simulink model "AuxClimaCtlMgr"  (R2022b) (continued)
== system AuxClimaCtlMgr == (continued)
  VFCPrkDrvgClimabyFragAct_VFCPrkDrvgClimabyFragAct : Outport  Port=66  OutDataTypeStr=Boolean  PortDimensions=1
  wires:
  AirClngSts_AirClngSts/1 -> AuxClimaCtlMgr_Runnable_sys/1(AirClngSts_AirClngSts_read)
  AirFragCh1AvlTi_AirFragCh1AvlTi/1 -> AuxClimaCtlMgr_Runnable_sys/2(AirFragCh1AvlTi_AirFragCh1AvlTi_read)
  AirFragCh1RunngSts_AirFragCh1RunngSts/1 -> AuxClimaCtlMgr_Runnable_sys/3(AirFragCh1RunngSts_AirFragCh1RunngSts_read)
  AirFragCh1Type_AirFragCh1Type/1 -> AuxClimaCtlMgr_Runnable_sys/4(AirFragCh1Type_AirFragCh1Type_read)
  AirFragCh2AvlTi_AirFragCh2AvlTi/1 -> AuxClimaCtlMgr_Runnable_sys/5(AirFragCh2AvlTi_AirFragCh2AvlTi_read)
  AirFragCh2RunngSts_AirFragCh2RunngSts/1 -> AuxClimaCtlMgr_Runnable_sys/6(AirFragCh2RunngSts_AirFragCh2RunngSts_read)
  AirFragCh2Type_AirFragCh2Type/1 -> AuxClimaCtlMgr_Runnable_sys/7(AirFragCh2Type_AirFragCh2Type_read)
  AirFragCh3AvlTi_AirFragCh3AvlTi/1 -> AuxClimaCtlMgr_Runnable_sys/8(AirFragCh3AvlTi_AirFragCh3AvlTi_read)
  AirFragCh3RunngSts_AirFragCh3RunngSts/1 -> AuxClimaCtlMgr_Runnable_sys/9(AirFragCh3RunngSts_AirFragCh3RunngSts_read)
  AirFragCh3Type_AirFragCh3Type/1 -> AuxClimaCtlMgr_Runnable_sys/10(AirFragCh3Type_AirFragCh3Type_read)
  AirFragCh4AvlTi_AirFragCh4AvlTi/1 -> AuxClimaCtlMgr_Runnable_sys/11(AirFragCh4AvlTi_AirFragCh4AvlTi_read)
  AirFragCh4RunngSts_AirFragCh4RunngSts/1 -> AuxClimaCtlMgr_Runnable_sys/12(AirFragCh4RunngSts_AirFragCh4RunngSts_read)
  AirFragCh4Type_AirFragCh4Type/1 -> AuxClimaCtlMgr_Runnable_sys/13(AirFragCh4Type_AirFragCh4Type_read)
  AirFragCh5AvlTi_AirFragCh5AvlTi/1 -> AuxClimaCtlMgr_Runnable_sys/14(AirFragCh5AvlTi_AirFragCh5AvlTi_read)
  AirFragCh5RunngSts_AirFragCh5RunngSts/1 -> AuxClimaCtlMgr_Runnable_sys/15(AirFragCh5RunngSts_AirFragCh5RunngSts_read)
  AirFragCh5Type_AirFragCh5Type/1 -> AuxClimaCtlMgr_Runnable_sys/16(AirFragCh5Type_AirFragCh5Type_read)
  AirFragChgSts_AirFragChgSts/1 -> AuxClimaCtlMgr_Runnable_sys/17(AirFragChgSts_AirFragChgSts_read)
  AirFragErrSts_AirFragErrSts/1 -> AuxClimaCtlMgr_Runnable_sys/18(AirFragErrSts_AirFragErrSts_read)
  AirFragInitSts_AirFragInitSts/1 -> AuxClimaCtlMgr_Runnable_sys/19(AirFragInitSts_AirFragInitSts_read)
  AirFragLvlRspn_AirFragLvlRspn/1 -> AuxClimaCtlMgr_Runnable_sys/20(AirFragLvlRspn_AirFragLvlRspn_read)
  AmbTEstimd_Ngcc_AmbTEstimd/1 -> AuxClimaCtlMgr_Runnable_sys/21(AmbTEstimd_Ngcc_AmbTEstimd_read)
  ClimaCfgForAirClng1_ClimaCfgForAirClng1/1 -> AuxClimaCtlMgr_Runnable_sys/22(ClimaCfgForAirClng1_ClimaCfgForAirClng1_read)
  ClimaCfgForAirFrag_ClimaCfgForAirFrag/1 -> AuxClimaCtlMgr_Runnable_sys/23(ClimaCfgForAirFrag_ClimaCfgForAirFrag_read)
  ClimaCfgForCds1_ClimaCfgForCds1/1 -> AuxClimaCtlMgr_Runnable_sys/24(ClimaCfgForCds1_ClimaCfgForCds1_read)
  ClimaCfgForVehElec1_ClimaCfgForVehElec1/1 -> AuxClimaCtlMgr_Runnable_sys/25(ClimaCfgForVehElec1_ClimaCfgForVehElec1_read)
  DrvrPfmncAlrmReq_DrvrPfmncAlrmReq/1 -> AuxClimaCtlMgr_Runnable_sys/26(DrvrPfmncAlrmReq_DrvrPfmncAlrmReq_read)
  DrvrPfmncAlrmReqFromDHU_DrvrPfmncAlrmReqFromDHU/1 -> AuxClimaCtlMgr_Runnable_sys/27(DrvrPfmncAlrmReqFromDHU_DrvrPfmncAlrmReqFromDHU_read)
  FragRefreshPopUpSucs_FragRefreshPopUpSucs/1 -> AuxClimaCtlMgr_Runnable_sys/28(FragRefreshPopUpSucs_FragRefreshPopUpSucs_read)
  FragRefrshAutSetg_FragRefrshAutSetg/1 -> AuxClimaCtlMgr_Runnable_sys/29(FragRefrshAutSetg_FragRefrshAutSetg_read)
  FrntHvacBlowerSts_FrntHvacBlowerSts/1 -> AuxClimaCtlMgr_Runnable_sys/30(FrntHvacBlowerSts_FrntHvacBlowerSts_read)
  HmiFragraModReq_HmiFragraModReq/1 -> AuxClimaCtlMgr_Runnable_sys/31(HmiFragraModReq_HmiFragraModReq_read)
  HmiPopUpResp_HmiPopUpResp/1 -> AuxClimaCtlMgr_Runnable_sys/32(HmiPopUpResp_HmiPopUpResp_read)
  InCarCo2HighWarnSucs_InCarCo2HighWarnSucs/1 -> AuxClimaCtlMgr_Runnable_sys/33(InCarCo2HighWarnSucs_InCarCo2HighWarnSucs_read)
  IntCo2CircElecErr_IntCo2CircElecErr/1 -> AuxClimaCtlMgr_Runnable_sys/34(IntCo2CircElecErr_IntCo2CircElecErr_read)
  IntCo2Conc_IntCo2Conc/1 -> AuxClimaCtlMgr_Runnable_sys/35(IntCo2Conc_IntCo2Conc_read)
  IntCo2fromExsrc_IntCo2fromExsrc/1 -> AuxClimaCtlMgr_Runnable_sys/36(IntCo2fromExsrc_IntCo2fromExsrc_read)
  IntCo2HvErr_IntCo2HvErr/1 -> AuxClimaCtlMgr_Runnable_sys/37(IntCo2HvErr_IntCo2HvErr_read)
  IntCo2LvErr_IntCo2LvErr/1 -> AuxClimaCtlMgr_Runnable_sys/38(IntCo2LvErr_IntCo2LvErr_read)
  IntCo2Lvl_IntCo2Lvl/1 -> AuxClimaCtlMgr_Runnable_sys/39(IntCo2Lvl_IntCo2Lvl_read)
  IntCo2RunMod_IntCo2RunMod/1 -> AuxClimaCtlMgr_Runnable_sys/40(IntCo2RunMod_IntCo2RunMod_read)
  IntCo2RunSts_IntCo2RunSts/1 -> AuxClimaCtlMgr_Runnable_sys/41(IntCo2RunSts_IntCo2RunSts_read)
  PwrAllwdForAirFrag_PwrAllwdForAirFrag/1 -> AuxClimaCtlMgr_Runnable_sys/42(PwrAllwdForAirFrag_PwrAllwdForAirFrag_read)
  VehModMngtGlbSafe1_Ngcc_VehModMngtGlbSafe1/1 -> AuxClimaCtlMgr_Runnable_sys/43(VehModMngtGlbSafe1_Ngcc_VehModMngtGlbSafe1_read)
  VehSunLoadTot_VehSunLoadTot/1 -> AuxClimaCtlMgr_Runnable_sys/44(VehSunLoadTot_VehSunLoadTot_read)
  CnctSrvFragChRatReq_CnctSrvFragChRatReq/1 -> AuxClimaCtlMgr_Runnable_sys/45(CnctSrvFragChRatReq_CnctSrvFragChRatReq_read)
  CnctSrvFragChRatReq_CnctSrvFragChRatReq_IsUpdated/1 -> AuxClimaCtlMgr_Runnable_sys/46(CnctSrvFragChRatReq_CnctSrvFragChRatReq_IsUpdated_read)
  CnctSrvFragLvlReq_CnctSrvFragLvlReq/1 -> AuxClimaCtlMgr_Runnable_sys/47(CnctSrvFragLvlReq_CnctSrvFragLvlReq_read)
  CnctSrvFragLvlReq_CnctSrvFragLvlReq_IsUpdated/1 -> AuxClimaCtlMgr_Runnable_sys/48(CnctSrvFragLvlReq_CnctSrvFragLvlReq_IsUpdated_read)
  HmiFragraChRatReq_HmiFragraChRatReq/1 -> AuxClimaCtlMgr_Runnable_sys/49(HmiFragraChRatReq_HmiFragraChRatReq_read)
  HmiFragraChRatReq_HmiFragraChRatReq_IsUpdated/1 -> AuxClimaCtlMgr_Runnable_sys/50(HmiFragraChRatReq_HmiFragraChRatReq_IsUpdated_read)
  HmiFragraLvlReq_HmiFragraLvlReq/1 -> AuxClimaCtlMgr_Runnable_sys/51(HmiFragraLvlReq_HmiFragraLvlReq_read)
  HmiFragraLvlReq_HmiFragraLvlReq_IsUpdated/1 -> AuxClimaCtlMgr_Runnable_sys/52(HmiFragraLvlReq_HmiFragraLvlReq_IsUpdated_read)
  ReqFragLvlTelm_ReqFragLvlTelm/1 -> AuxClimaCtlMgr_Runnable_sys/53(ReqFragLvlTelm_ReqFragLvlTelm_read)
  ReqFragLvlTelm_ReqFragLvlTelm_IsUpdated/1 -> AuxClimaCtlMgr_Runnable_sys/54(ReqFragLvlTelm_ReqFragLvlTelm_IsUpdated_read)
  TelmAirFragTasteReq_TelmAirFragTasteReq/1 -> AuxClimaCtlMgr_Runnable_sys/55(TelmAirFragTasteReq_TelmAirFragTasteReq_read)
  TelmAirFragTasteReq_TelmAirFragTasteReq_IsUpdated/1 -> AuxClimaCtlMgr_Runnable_sys/56(TelmAirFragTasteReq_TelmAirFragTasteReq_IsUpdated_read)
  AACAirClngStsNgcc_AACAirClngStsNgcc/1 -> AuxClimaCtlMgr_Runnable_sys/57(AACAirClngStsNgcc_AACAirClngStsNgcc_read)
  AFUFragraCh1AvlTiPrecNgcc_AFUFragraCh1AvlTiPrecNgcc/1 -> AuxClimaCtlMgr_Runnable_sys/58(AFUFragraCh1AvlTiPrecNgcc_AFUFragraCh1AvlTiPrecNgcc_read)
  AFUFragraCh1AvlTiPrecNgcc_AFUFragraCh1AvlTiPrecNgcc_IsUpdated/1 -> AuxClimaCtlMgr_Runnable_sys/59(AFUFragraCh1AvlTiPrecNgcc_AFUFragraCh1AvlTiPrecNgcc_IsUpdated_read)
  AFUFragraCh2AvlTiPrecNgcc_AFUFragraCh2AvlTiPrecNgcc/1 -> AuxClimaCtlMgr_Runnable_sys/60(AFUFragraCh2AvlTiPrecNgcc_AFUFragraCh2AvlTiPrecNgcc_read)
  AFUFragraCh2AvlTiPrecNgcc_AFUFragraCh2AvlTiPrecNgcc_IsUpdated/1 -> AuxClimaCtlMgr_Runnable_sys/61(AFUFragraCh2AvlTiPrecNgcc_AFUFragraCh2AvlTiPrecNgcc_IsUpdated_read)
  AFUFragraCh3AvlTiPrecNgcc_AFUFragraCh3AvlTiPrecNgcc/1 -> AuxClimaCtlMgr_Runnable_sys/62(AFUFragraCh3AvlTiPrecNgcc_AFUFragraCh3AvlTiPrecNgcc_read)
  AFUFragraCh3AvlTiPrecNgcc_AFUFragraCh3AvlTiPrecNgcc_IsUpdated/1 -> AuxClimaCtlMgr_Runnable_sys/63(AFUFragraCh3AvlTiPrecNgcc_AFUFragraCh3AvlTiPrecNgcc_IsUpdated_read)
  AFUFragraCh4AvlTiPrecNgcc_AFUFragraCh4AvlTiPrecNgcc/1 -> AuxClimaCtlMgr_Runnable_sys/64(AFUFragraCh4AvlTiPrecNgcc_AFUFragraCh4AvlTiPrecNgcc_read)
  AFUFragraCh4AvlTiPrecNgcc_AFUFragraCh4AvlTiPrecNgcc_IsUpdated/1 -> AuxClimaCtlMgr_Runnable_sys/65(AFUFragraCh4AvlTiPrecNgcc_AFUFragraCh4AvlTiPrecNgcc_IsUpdated_read)
  AFUFragraCh5AvlTiPrecNgcc_AFUFragraCh5AvlTiPrecNgcc/1 -> AuxClimaCtlMgr_Runnable_sys/66(AFUFragraCh5AvlTiPrecNgcc_AFUFragraCh5AvlTiPrecNgcc_read)
  AFUFragraCh5AvlTiPrecNgcc_AFUFragraCh5AvlTiPrecNgcc_IsUpdated/1 -> AuxClimaCtlMgr_Runnable_sys/67(AFUFragraCh5AvlTiPrecNgcc_AFUFragraCh5AvlTiPrecNgcc_IsUpdated_read)
  AirClngReq_AirClngReq/1 -> AuxClimaCtlMgr_Runnable_sys/68(AirClngReq_AirClngReq_read)
  AirClngReq_AirClngReq_IsUpdated/1 -> AuxClimaCtlMgr_Runnable_sys/69(AirClngReq_AirClngReq_IsUpdated_read)
  AirFragCh1OvrdDyNgcc_AirFragCh1OvrdDyNgcc/1 -> AuxClimaCtlMgr_Runnable_sys/70(AirFragCh1OvrdDyNgcc_AirFragCh1OvrdDyNgcc_read)
  AirFragCh2OvrdDyNgcc_AirFragCh2OvrdDyNgcc/1 -> AuxClimaCtlMgr_Runnable_sys/71(AirFragCh2OvrdDyNgcc_AirFragCh2OvrdDyNgcc_read)
  AirFragCh3OvrdDyNgcc_AirFragCh3OvrdDyNgcc/1 -> AuxClimaCtlMgr_Runnable_sys/72(AirFragCh3OvrdDyNgcc_AirFragCh3OvrdDyNgcc_read)
  AirFragCh4OvrdDyNgcc_AirFragCh4OvrdDyNgcc/1 -> AuxClimaCtlMgr_Runnable_sys/73(AirFragCh4OvrdDyNgcc_AirFragCh4OvrdDyNgcc_read)
  AirFragCh5OvrdDyNgcc_AirFragCh5OvrdDyNgcc/1 -> AuxClimaCtlMgr_Runnable_sys/74(AirFragCh5OvrdDyNgcc_AirFragCh5OvrdDyNgcc_read)
  AirSrcStsFbNgcc_AirSrcStsFbNgcc/1 -> AuxClimaCtlMgr_Runnable_sys/75(AirSrcStsFbNgcc_AirSrcStsFbNgcc_read)
  AltitudeInfromNgcc_AltitudeInfromNgcc/1 -> AuxClimaCtlMgr_Runnable_sys/76(AltitudeInfromNgcc_AltitudeInfromNgcc_read)
  ClimaCfgForAAC_ClimaCfgForAAC/1 -> AuxClimaCtlMgr_Runnable_sys/77(ClimaCfgForAAC_ClimaCfgForAAC_read)
  ClimaCfgForAirClnrAdv1_ClimaCfgForAirClnrAdv1/1 -> AuxClimaCtlMgr_Runnable_sys/78(ClimaCfgForAirClnrAdv1_ClimaCfgForAirClnrAdv1_read)
  ClimaCfgForCabinOxgenSupply_ClimaCfgForCabinOxgenSupply/1 -> AuxClimaCtlMgr_Runnable_sys/79(ClimaCfgForCabinOxgenSupply_ClimaCfgForCabinOxgenSupply_read)
  ClimaCfgForPrpsnTyp_ClimaCfgForPrpsnTyp/1 -> AuxClimaCtlMgr_Runnable_sys/80(ClimaCfgForPrpsnTyp_ClimaCfgForPrpsnTyp_read)
  ClimaCfgForRoofHatch_ClimaCfgForRoofHatch/1 -> AuxClimaCtlMgr_Runnable_sys/81(ClimaCfgForRoofHatch_ClimaCfgForRoofHatch_read)
  CmptmtOxygenConcQFNgcc_CmptmtOxygenConcQFNgcc/1 -> AuxClimaCtlMgr_Runnable_sys/82(CmptmtOxygenConcQFNgcc_CmptmtOxygenConcQFNgcc_read)
  CmptmtOxygenConcVluNgcc_CmptmtOxygenConcVluNgcc/1 -> AuxClimaCtlMgr_Runnable_sys/83(CmptmtOxygenConcVluNgcc_CmptmtOxygenConcVluNgcc_read)
  DispHvBattLvlOfChrgNgcc_DispHvBattLvlOfChrgNgcc/1 -> AuxClimaCtlMgr_Runnable_sys/84(DispHvBattLvlOfChrgNgcc_DispHvBattLvlOfChrgNgcc_read)
  DoorDrvrReSts_DoorDrvrReSts/1 -> AuxClimaCtlMgr_Runnable_sys/85(DoorDrvrReSts_DoorDrvrReSts_read)
  DoorDrvrSts_Ngcc_DoorDrvrSts/1 -> AuxClimaCtlMgr_Runnable_sys/86(DoorDrvrSts_Ngcc_DoorDrvrSts_read)
  DoorPassReSts_DoorPassReSts/1 -> AuxClimaCtlMgr_Runnable_sys/87(DoorPassReSts_DoorPassReSts_read)
  DoorPassSts_Ngcc_DoorPassSts/1 -> AuxClimaCtlMgr_Runnable_sys/88(DoorPassSts_Ngcc_DoorPassSts_read)
  DrvrVentLeClsdFlag_DrvrVentLeClsdFlag/1 -> AuxClimaCtlMgr_Runnable_sys/89(DrvrVentLeClsdFlag_DrvrVentLeClsdFlag_read)
  DrvrVentRiClsdFlag_DrvrVentRiClsdFlag/1 -> AuxClimaCtlMgr_Runnable_sys/90(DrvrVentRiClsdFlag_DrvrVentRiClsdFlag_read)
  HmiAutOxySetNgcc_HmiAutOxySetNgcc/1 -> AuxClimaCtlMgr_Runnable_sys/91(HmiAutOxySetNgcc_HmiAutOxySetNgcc_read)
  HmiOxyinCarSwtNgcc_HmiOxyinCarSwtNgcc/1 -> AuxClimaCtlMgr_Runnable_sys/92(HmiOxyinCarSwtNgcc_HmiOxyinCarSwtNgcc_read)
  HvacRecircAct_Ngcc_HvacRecircAct/1 -> AuxClimaCtlMgr_Runnable_sys/93(HvacRecircAct_Ngcc_HvacRecircAct_read)
  HvSysRlySts_HvSysRlySts/1 -> AuxClimaCtlMgr_Runnable_sys/94(HvSysRlySts_HvSysRlySts_read)
  InflateAirSrcSwtNgcc_InflateAirSrcSwtNgcc/1 -> AuxClimaCtlMgr_Runnable_sys/95(InflateAirSrcSwtNgcc_InflateAirSrcSwtNgcc_read)
  OGMAirSrcReq_OGMAirSrcReq/1 -> AuxClimaCtlMgr_Runnable_sys/96(OGMAirSrcReq_OGMAirSrcReq_read)
  OGMAvlSts_OGMAvlSts/1 -> AuxClimaCtlMgr_Runnable_sys/97(OGMAvlSts_OGMAvlSts_read)
  OGMIntErrNgcc_OGMIntErrNgcc/1 -> AuxClimaCtlMgr_Runnable_sys/98(OGMIntErrNgcc_OGMIntErrNgcc_read)
  OGMOvrVoltRangErrNgcc_OGMOvrVoltRangErrNgcc/1 -> AuxClimaCtlMgr_Runnable_sys/99(OGMOvrVoltRangErrNgcc_OGMOvrVoltRangErrNgcc_read)
  OGMReq_OGMReq/1 -> AuxClimaCtlMgr_Runnable_sys/100(OGMReq_OGMReq_read)
  OGMTempErrNgcc_OGMTempErrNgcc/1 -> AuxClimaCtlMgr_Runnable_sys/101(OGMTempErrNgcc_OGMTempErrNgcc_read)
  OGMUndVoltRangErrNgcc_OGMUndVoltRangErrNgcc/1 -> AuxClimaCtlMgr_Runnable_sys/102(OGMUndVoltRangErrNgcc_OGMUndVoltRangErrNgcc_read)
  OxygenConcSnsrElecErrNgcc_OxygenConcSnsrElecErrNgcc/1 -> AuxClimaCtlMgr_Runnable_sys/103(OxygenConcSnsrElecErrNgcc_OxygenConcSnsrElecErrNgcc_read)
  OxygenConcSnsrIntErrNgcc_OxygenConcSnsrIntErrNgcc/1 -> AuxClimaCtlMgr_Runnable_sys/104(OxygenConcSnsrIntErrNgcc_OxygenConcSnsrIntErrNgcc_read)
  OxygenConcSnsrOvrVoltRangErrNgcc_OxygenConcSnsrOvrVoltRangErrNgcc/1 -> AuxClimaCtlMgr_Runnable_sys/105(OxygenConcSnsrOvrVoltRangErrNgcc_OxygenConcSnsrOvrVoltRangErrNgcc_read)
  OxygenConcSnsrStsNgcc_OxygenConcSnsrStsNgcc/1 -> AuxClimaCtlMgr_Runnable_sys/106(OxygenConcSnsrStsNgcc_OxygenConcSnsrStsNgcc_read)
  OxygenConcSnsrTempErrNgcc_OxygenConcSnsrTempErrNgcc/1 -> AuxClimaCtlMgr_Runnable_sys/107(OxygenConcSnsrTempErrNgcc_OxygenConcSnsrTempErrNgcc_read)
  OxygenConcSnsrUndVoltRangErrNgcc_OxygenConcSnsrUndVoltRangErrNgcc/1 -> AuxClimaCtlMgr_Runnable_sys/108(OxygenConcSnsrUndVoltRangErrNgcc_OxygenConcSnsrUndVoltRangErrNgcc_read)
  OxygenGenrErrInfoNgcc_OxygenGenrErrInfoNgcc/1 -> AuxClimaCtlMgr_Runnable_sys/109(OxygenGenrErrInfoNgcc_OxygenGenrErrInfoNgcc_read)
  OxygenSplyAvlStsNgcc_OxygenSplyAvlStsNgcc/1 -> AuxClimaCtlMgr_Runnable_sys/110(OxygenSplyAvlStsNgcc_OxygenSplyAvlStsNgcc_read)
  OxygenSplyErrNgcc_OxygenSplyErrNgcc/1 -> AuxClimaCtlMgr_Runnable_sys/111(OxygenSplyErrNgcc_OxygenSplyErrNgcc_read)
  OxygenSplyPwrAllwdNgcc_OxygenSplyPwrAllwdNgcc/1 -> AuxClimaCtlMgr_Runnable_sys/112(OxygenSplyPwrAllwdNgcc_OxygenSplyPwrAllwdNgcc_read)
  PassVentLeClsdFlag_PassVentLeClsdFlag/1 -> AuxClimaCtlMgr_Runnable_sys/113(PassVentLeClsdFlag_PassVentLeClsdFlag_read)
  PassVentRiClsdFlag_PassVentRiClsdFlag/1 -> AuxClimaCtlMgr_Runnable_sys/114(PassVentRiClsdFlag_PassVentRiClsdFlag_read)
  RemOxygenSplyActv_RemOxygenSplyActv/1 -> AuxClimaCtlMgr_Runnable_sys/115(RemOxygenSplyActv_RemOxygenSplyActv_read)
  SunRoofPosnSts_Ngcc_SunRoofPosnSts/1 -> AuxClimaCtlMgr_Runnable_sys/116(SunRoofPosnSts_Ngcc_SunRoofPosnSts_read)
  TelmOxygenValReqNgcc_TelmOxygenValReqNgcc/1 -> AuxClimaCtlMgr_Runnable_sys/117(TelmOxygenValReqNgcc_TelmOxygenValReqNgcc_read)
  TiAndDateIndcn_TiAndDateIndcn/1 -> AuxClimaCtlMgr_Runnable_sys/118(TiAndDateIndcn_TiAndDateIndcn_read)
  TrSts_Ngcc_TrSts/1 -> AuxClimaCtlMgr_Runnable_sys/119(TrSts_Ngcc_TrSts_read)
  TrSts_Ngcc_TrSts_ErrorStatus/1 -> AuxClimaCtlMgr_Runnable_sys/120(TrSts_Ngcc_TrSts_ErrorStatus_read)
  WinPosnStsAtDrvrReNgcc_WinPosnStsAtDrvrReNgcc/1 -> AuxClimaCtlMgr_Runnable_sys/121(WinPosnStsAtDrvrReNgcc_WinPosnStsAtDrvrReNgcc_read)
  WinPosnStsAtPassReNgcc_WinPosnStsAtPassRe/1 -> AuxClimaCtlMgr_Runnable_sys/122(WinPosnStsAtPassReNgcc_WinPosnStsAtPassRe_read)
  WinPosnStsDrv_WinPosnStsAtDrv/1 -> AuxClimaCtlMgr_Runnable_sys/123(WinPosnStsDrv_WinPosnStsAtDrv_read)
  WinPosnStsPass_WinPosnStsAtPass/1 -> AuxClimaCtlMgr_Runnable_sys/124(WinPosnStsPass_WinPosnStsAtPass_read)
  AuxClimaCtlMgr_Runnable_sys/1(AACAirClngActnNgcc_AACAirClngActnNgcc_write) -> AACAirClngActnNgcc_AACAirClngActnNgcc/1
  AuxClimaCtlMgr_Runnable_sys/2(AirSrcReqNgcc_AirSrcReqNgcc_write) -> AirSrcReqNgcc_AirSrcReqNgcc/1
  AuxClimaCtlMgr_Runnable_sys/3(AutOxygenSplyStsFrmClimaNgcc_AutOxygenSplyStsFrmClimaNgcc_write) -> AutOxygenSplyStsFrmClimaNgcc_AutOxygenSplyStsFrmClimaNgcc/1
  AuxClimaCtlMgr_Runnable_sys/4(CcmAACAirClngStsNgcc_CcmAACAirClngStsNgcc_write) -> CcmAACAirClngStsNgcc_CcmAACAirClngStsNgcc/1
  AuxClimaCtlMgr_Runnable_sys/5(CCMFragCh1OvrdDyNgcc_CCMFragCh1OvrdDyNgcc_write) -> CCMFragCh1OvrdDyNgcc_CCMFragCh1OvrdDyNgcc/1
  AuxClimaCtlMgr_Runnable_sys/6(CCMFragCh2OvrdDyNgcc_CCMFragCh2OvrdDyNgcc_write) -> CCMFragCh2OvrdDyNgcc_CCMFragCh2OvrdDyNgcc/1
  AuxClimaCtlMgr_Runnable_sys/7(CCMFragCh3OvrdDyNgcc_CCMFragCh3OvrdDyNgcc_write) -> CCMFragCh3OvrdDyNgcc_CCMFragCh3OvrdDyNgcc/1
  AuxClimaCtlMgr_Runnable_sys/8(CCMFragCh4OvrdDyNgcc_CCMFragCh4OvrdDyNgcc_write) -> CCMFragCh4OvrdDyNgcc_CCMFragCh4OvrdDyNgcc/1
  AuxClimaCtlMgr_Runnable_sys/9(CCMFragCh5OvrdDyNgcc_CCMFragCh5OvrdDyNgcc_write) -> CCMFragCh5OvrdDyNgcc_CCMFragCh5OvrdDyNgcc/1
  AuxClimaCtlMgr_Runnable_sys/10(CCMFragraCh1AvlTiPrecNgcc_CCMFragraCh1AvlTiPrecNgcc_write) -> CCMFragraCh1AvlTiPrecNgcc_CCMFragraCh1AvlTiPrecNgcc/1
  AuxClimaCtlMgr_Runnable_sys/11(CCMFragraCh2AvlTiPrecNgcc_CCMFragraCh2AvlTiPrecNgcc_write) -> CCMFragraCh2AvlTiPrecNgcc_CCMFragraCh2AvlTiPrecNgcc/1
  AuxClimaCtlMgr_Runnable_sys/12(CCMFragraCh3AvlTiPrecNgcc_CCMFragraCh3AvlTiPrecNgcc_write) -> CCMFragraCh3AvlTiPrecNgcc_CCMFragraCh3AvlTiPrecNgcc/1
  AuxClimaCtlMgr_Runnable_sys/13(CCMFragraCh4AvlTiPrecNgcc_CCMFragraCh4AvlTiPrecNgcc_write) -> CCMFragraCh4AvlTiPrecNgcc_CCMFragraCh4AvlTiPrecNgcc/1
  AuxClimaCtlMgr_Runnable_sys/14(CCMFragraCh5AvlTiPrecNgcc_CCMFragraCh5AvlTiPrecNgcc_write) -> CCMFragraCh5AvlTiPrecNgcc_CCMFragraCh5AvlTiPrecNgcc/1
  AuxClimaCtlMgr_Runnable_sys/15(CmptmtOxygenConcLvlFrmClimaNgcc_CmptmtOxygenConcLvlFrmClimaNgcc_write) -> CmptmtOxygenConcLvlFrmClimaNgcc_CmptmtOxygenConcLvlFrmClimaNgcc/1
  AuxClimaCtlMgr_Runnable_sys/16(CmptmtOxygenConcVluFrmClimaNgcc_CmptmtOxygenConcVluFrmClimaNgcc_write) -> CmptmtOxygenConcVluFrmClimaNgcc_CmptmtOxygenConcVluFrmClimaNgcc/1
  AuxClimaCtlMgr_Runnable_sys/17(OSMRecircRatReq_OSMRecircRatReq_write) -> OSMRecircRatReq_OSMRecircRatReq/1
  AuxClimaCtlMgr_Runnable_sys/18(OxygenConcSnsrActnReqNgcc_OxygenConcSnsrActnReqNgcc_write) -> OxygenConcSnsrActnReqNgcc_OxygenConcSnsrActnReqNgcc/1
  AuxClimaCtlMgr_Runnable_sys/19(OxygenConcSnsrStsFrmClimaNgcc_OxygenConcSnsrStsFrmClimaNgcc_write) -> OxygenConcSnsrStsFrmClimaNgcc_OxygenConcSnsrStsFrmClimaNgcc/1
  AuxClimaCtlMgr_Runnable_sys/20(OxygenSplyActPwrCns_OxygenSplyActPwrCns_write) -> OxygenSplyActPwrCns_OxygenSplyActPwrCns/1
  AuxClimaCtlMgr_Runnable_sys/21(OxygenSplyAvlStsFrmClimaNgcc_OxygenSplyAvlStsFrmClimaNgcc_write) -> OxygenSplyAvlStsFrmClimaNgcc_OxygenSplyAvlStsFrmClimaNgcc/1
  AuxClimaCtlMgr_Runnable_sys/22(OxygenSplyDesPwrCns_OxygenSplyDesPwrCns_write) -> OxygenSplyDesPwrCns_OxygenSplyDesPwrCns/1
  AuxClimaCtlMgr_Runnable_sys/23(OxygenSplyErrFrmClimaNgcc_OxygenSplyErrFrmClimaNgcc_write) -> OxygenSplyErrFrmClimaNgcc_OxygenSplyErrFrmClimaNgcc/1
  AuxClimaCtlMgr_Runnable_sys/24(OxygenSplyReqFaildNgcc_OxygenSplyReqFaildNgcc_write) -> OxygenSplyReqFaildNgcc_OxygenSplyReqFaildNgcc/1
  AuxClimaCtlMgr_Runnable_sys/25(OxygenSplyReqNgcc_OxygenSplyReqNgcc_write) -> OxygenSplyReqNgcc_OxygenSplyReqNgcc/1
  AuxClimaCtlMgr_Runnable_sys/26(OxygenSplyVoiceFbNgcc_OxygenSplyVoiceFbNgcc_write) -> OxygenSplyVoiceFbNgcc_OxygenSplyVoiceFbNgcc/1
  AuxClimaCtlMgr_Runnable_sys/27(PopUpReqFromOxygenSply_PopUpReqFromOxygenSply_write) -> PopUpReqFromOxygenSply_PopUpReqFromOxygenSply/1
  AuxClimaCtlMgr_Runnable_sys/28(VFCPrkDrvgClimabyOxygenSplyCtrl_VFCPrkDrvgClimabyOxygenSplyCtrl_write) -> VFCPrkDrvgClimabyOxygenSplyCtrl_VFCPrkDrvgClimabyOxygenSplyCtrl/1
  AuxClimaCtlMgr_Runnable_sys/29(AirClngActn_AirClngActn_write) -> AirClngActn_AirClngActn/1
  AuxClimaCtlMgr_Runnable_sys/30(AirFragActEnad_AirFragActEnad_write) -> AirFragActEnad_AirFragActEnad/1
  AuxClimaCtlMgr_Runnable_sys/31(AirFragCh1_AirFragCh1_write) -> AirFragCh1_AirFragCh1/1
  AuxClimaCtlMgr_Runnable_sys/32(AirFragCh2_AirFragCh2_write) -> AirFragCh2_AirFragCh2/1
  AuxClimaCtlMgr_Runnable_sys/33(AirFragCh3_AirFragCh3_write) -> AirFragCh3_AirFragCh3/1
  AuxClimaCtlMgr_Runnable_sys/34(AirFragCh4_AirFragCh4_write) -> AirFragCh4_AirFragCh4/1
  AuxClimaCtlMgr_Runnable_sys/35(AirFragCh5_AirFragCh5_write) -> AirFragCh5_AirFragCh5/1
  AuxClimaCtlMgr_Runnable_sys/36(AirFragConcReq_AirFragConcReq_write) -> AirFragConcReq_AirFragConcReq/1
  AuxClimaCtlMgr_Runnable_sys/37(AirFragPwrCnsAct_AirFragPwrCnsAct_write) -> AirFragPwrCnsAct_AirFragPwrCnsAct/1
  AuxClimaCtlMgr_Runnable_sys/38(AirFragPwrCnsDes_AirFragPwrCnsDes_write) -> AirFragPwrCnsDes_AirFragPwrCnsDes/1
  AuxClimaCtlMgr_Runnable_sys/39(CcmAirClngSts_CcmAirClngSts_write) -> CcmAirClngSts_CcmAirClngSts/1
  AuxClimaCtlMgr_Runnable_sys/40(CcmIntCo2Conc_CcmIntCo2Conc_write) -> CcmIntCo2Conc_CcmIntCo2Conc/1
  AuxClimaCtlMgr_Runnable_sys/41(CcmIntCo2Lvl_CcmIntCo2Lvl_write) -> CcmIntCo2Lvl_CcmIntCo2Lvl/1
  AuxClimaCtlMgr_Runnable_sys/42(DrvgCDSClimaOnFlag_DrvgCDSClimaOnFlag_write) -> DrvgCDSClimaOnFlag_DrvgCDSClimaOnFlag/1
  AuxClimaCtlMgr_Runnable_sys/43(DrvgCDSRecirReq_DrvgCDSRecirReq_write) -> DrvgCDSRecirReq_DrvgCDSRecirReq/1
  AuxClimaCtlMgr_Runnable_sys/44(FragCh1Id_FragCh1Id_write) -> FragCh1Id_FragCh1Id/1
  AuxClimaCtlMgr_Runnable_sys/45(FragCh1UseUpWrn_FragCh1UseUpWrn_write) -> FragCh1UseUpWrn_FragCh1UseUpWrn/1
  AuxClimaCtlMgr_Runnable_sys/46(FragCh2Id_FragCh2Id_write) -> FragCh2Id_FragCh2Id/1
  AuxClimaCtlMgr_Runnable_sys/47(FragCh2UseUpWrn_FragCh2UseUpWrn_write) -> FragCh2UseUpWrn_FragCh2UseUpWrn/1
  AuxClimaCtlMgr_Runnable_sys/48(FragCh3Id_FragCh3Id_write) -> FragCh3Id_FragCh3Id/1
  AuxClimaCtlMgr_Runnable_sys/49(FragCh3UseUpWrn_FragCh3UseUpWrn_write) -> FragCh3UseUpWrn_FragCh3UseUpWrn/1
  AuxClimaCtlMgr_Runnable_sys/50(FragCh4Id_FragCh4Id_write) -> FragCh4Id_FragCh4Id/1
  AuxClimaCtlMgr_Runnable_sys/51(FragCh4UseUpWrn_FragCh4UseUpWrn_write) -> FragCh4UseUpWrn_FragCh4UseUpWrn/1
  AuxClimaCtlMgr_Runnable_sys/52(FragCh5Id_FragCh5Id_write) -> FragCh5Id_FragCh5Id/1
  AuxClimaCtlMgr_Runnable_sys/53(FragCh5UseUpWrn_FragCh5UseUpWrn_write) -> FragCh5UseUpWrn_FragCh5UseUpWrn/1
  AuxClimaCtlMgr_Runnable_sys/54(FragLvlFrmClima_FragLvlFrmClima_write) -> FragLvlFrmClima_FragLvlFrmClima/1
  AuxClimaCtlMgr_Runnable_sys/55(FragRefreshPopUpByAux_FragRefreshPopUpByAux_write) -> FragRefreshPopUpByAux_FragRefreshPopUpByAux/1
  FragStsFrmClima_FragStsFrmClima_merge/1 -> FragStsFrmClima_FragStsFrmClima/1
  AuxClimaCtlMgr_Runnable_sys/57(InCarCo2HighWarnByCDS_InCarCo2HighWarnByCDS_write) -> InCarCo2HighWarnByCDS_InCarCo2HighWarnByCDS/1
  IntCo2AmbSnsrTmp_IntCo2AmbSnsrTmp_merge/1 -> IntCo2AmbSnsrTmp_IntCo2AmbSnsrTmp/1
  AuxClimaCtlMgr_Runnable_sys/59(IntCo2SnsrActv_IntCo2SnsrActv_write) -> IntCo2SnsrActv_IntCo2SnsrActv/1
  AuxClimaCtlMgr_Runnable_sys/60(IntCo2SnsrActvDrvgFlag_IntCo2SnsrActvDrvgFlag_write) -> IntCo2SnsrActvDrvgFlag_IntCo2SnsrActvDrvgFlag/1
  AuxClimaCtlMgr_Runnable_sys/61(IntCo2SolarIntenLe_IntCo2SolarIntenLe_write) -> IntCo2SolarIntenLe_IntCo2SolarIntenLe/1
  AuxClimaCtlMgr_Runnable_sys/62(IntCo2SolarIntenRi_IntCo2SolarIntenRi_write) -> IntCo2SolarIntenRi_IntCo2SolarIntenRi/1
  AuxClimaCtlMgr_Runnable_sys/63(RlyCmftForFragReq_RlyCmftForFragReq_write) -> RlyCmftForFragReq_RlyCmftForFragReq/1
  AuxClimaCtlMgr_Runnable_sys/64(SunRoofOpnPopUpForHmi_SunRoofOpnPopUpForHmi_write) -> SunRoofOpnPopUpForHmi_SunRoofOpnPopUpForHmi/1
  AuxClimaCtlMgr_Runnable_sys/65(VFCInfoPushInCarCDSMon_VFCInfoPushInCarCDSMon_write) -> VFCInfoPushInCarCDSMon_VFCInfoPushInCarCDSMon/1
  AuxClimaCtlMgr_Runnable_sys/66(VFCPrkDrvgClimabyFragAct_VFCPrkDrvgClimabyFragAct_write) -> VFCPrkDrvgClimabyFragAct_VFCPrkDrvgClimabyFragAct/1
  AuxClimaCtlMgr_Runnable_sys/58(IntCo2AmbSnsrTmp_IntCo2AmbSnsrTmp_write) -> [IntCo2AmbSnsrTmp_IntCo2AmbSnsrTmp_merge_Tag]
  [IntCo2AmbSnsrTmp_IntCo2AmbSnsrTmp_merge_Tag] -> IntCo2AmbSnsrTmp_IntCo2AmbSnsrTmp_merge/2
  AuxClimaCtlMgr_Init/2(IntCo2AmbSnsrTmp_IntCo2AmbSnsrTmp_write) -> [IntCo2AmbSnsrTmp_IntCo2AmbSnsrTmp_merge_Tag1]
  [IntCo2AmbSnsrTmp_IntCo2AmbSnsrTmp_merge_Tag1] -> IntCo2AmbSnsrTmp_IntCo2AmbSnsrTmp_merge/1
  AuxClimaCtlMgr_Runnable_sys/56(FragStsFrmClima_FragStsFrmClima_write) -> [FragStsFrmClima_FragStsFrmClima_merge_Tag]
  [FragStsFrmClima_FragStsFrmClima_merge_Tag] -> FragStsFrmClima_FragStsFrmClima_merge/2
  AuxClimaCtlMgr_Init/1(FragStsFrmClima_FragStsFrmClima_write) -> [FragStsFrmClima_FragStsFrmClima_merge_Tag1]
  [FragStsFrmClima_FragStsFrmClima_merge_Tag1] -> FragStsFrmClima_FragStsFrmClima_merge/1
  AuxClimaCtlMgr_Runnable/1 -> AuxClimaCtlMgr_Runnable_sys/Trigger

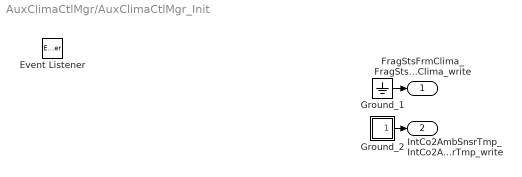
== system AuxClimaCtlMgr/AuxClimaCtlMgr_Init ==
  Event Listener : EventListener  EventName=reset
  Ground_1 : Ground
  Ground_2 : Subsystem
  FragStsFrmClima_FragStsFrmClima_write : Outport
  IntCo2AmbSnsrTmp_IntCo2AmbSnsrTmp_write : Outport  Port=2
  wires:
  Ground_1/1 -> FragStsFrmClima_FragStsFrmClima_write/1
  Ground_2/1(Out1) -> IntCo2AmbSnsrTmp_IntCo2AmbSnsrTmp_write/1
